simulink model "AcasXuClosedLoop_all_comp"  (Simulink 10.0)
settings: Solver=VariableStepAuto  StartTime=0.0  StopTime=20  RelTol=1e-3
libraries: neural x14040, simulink x2
(part 32 of 534)

== system AcasXuClosedLoop_all_comp/networks/Networks_m_7/AcasXu_5_7/Process Output 1 ==
  a : Inport  PortDimensions=5
      mask Display: e = 0.06;c1 = [120 230 180]/255;c2 = ([120 230 180]+0)/(256+128);c3 = ([120 230 180]+128)/(256+128);patch([0 1 1 0],[0 0 1 1],c1);patch([0 1 1 1-e 1-e 0+e],[0 0 1 1-e 0+e 0+e],c2);patch([0 0 1 1-e 0+e 0+e],[0 1 1 1-e 1-e 0+e],c3);plot([0 1 1 0 0],[0 0 1 1 0]);
  y : Outport  PortDimensions=5
      mask Display: e = 0.06;c1 = [120 230 180]/255;c2 = ([120 230 180]+0)/(256+128);c3 = ([120 230 180]+128)/(256+128);patch([0 1 1 0],[0 0 1 1],c1);patch([0 1 1 1-e 1-e 0+e],[0 0 1 1-e 0+e 0+e],c2);patch([0 0 1 1-e 0+e 0+e],[0 1 1 1-e 1-e 0+e],c3);plot([0 1 1 0 0],[0 0 1 1 0]);
  wires:
  a/1 -> y/1

== system AcasXuClosedLoop_all_comp/networks/Networks_m_8/AcasXu_1_8/Layer 1 ==
  "p{1}" : Inport  PortDimensions=5
      mask Display: e = 0.06;c1 = [120 230 180]/255;c2 = ([120 230 180]+0)/(256+128);c3 = ([120 230 180]+128)/(256+128);patch([0 1 1 0],[0 0 1 1],c1);patch([0 1 1 1-e 1-e 0+e],[0 0 1 1-e 0+e 0+e],c2);patch([0 0 1 1-e 0+e 0+e],[0 1 1 1-e 1-e 0+e],c3);plot([0 1 1 0 0],[0 0 1 1 0]);
  Delays 1 : Subsystem
      mask Display: e = 0.06;c1 = [120 150 230]/255;c2 = ([120 150 230]+0)/383;c3 = ([120 150 230]+128)/383;patch([0 1 1 0],[0 0 1 1],c1);patch([0 1 1 1-e 1-e 0+e],[0 0 1 1-e 0+e 0+e],c2);patch([0 0 1 1-e 0+e 0+e],[0 1 1 1-e 1-e 0+e],c3);plot([0 1 1 0 0],[0 0 1 1 0]);disp('0');
  "IW{1,1}" : Subsystem
      mask Display: e = 0.06;c1 = [120 150 230]/255;c2 = ([120 150 230]+0)/383;c3 = ([120 150 230]+128)/383;patch([0 1 1 0],[0 0 1 1],c1);patch([0 1 1 1-e 1-e 0+e],[0 0 1 1-e 0+e 0+e],c2);patch([0 0 1 1-e 0+e 0+e],[0 1 1 1-e 1-e 0+e],c3);plot([0 1 1 0 0],[0 0 1 1 0]);disp('W');
  "b{1}" : Constant  Value=[0.061786899999999998767119535614256164990365505218505859375;-0.11233100000000000029398705692074145190417766571044921875;0.0576498000000000010434320074637071229517459869384765625;-0.0022952799999999998030453252084726045723073184490203857421875;-0.020411800000000000776534392343819490633904933929443359375;-0.033371199999999996699262538868424599058926105499267578125;-0.0168530999999999993310684232028506812639534473419189453125;0.285644999999999982254195174391497857868671417236328125;0.074333399999999993923438523779623210430145263671875;-0.0687670999999999976726172690177918411791324615478515625;-0.055837400000000002309175073378355591557919979095458984375;-0.198448000000000013276491017677471973001956939697265625;0.2412819999999999964757080306299030780792236328125;-0.02656630000000000102478026065000449307262897491455078125;-0.15976000000000001310951347477384842932224273681640625;0.06498429999999999484572299479623325169086456298828125;-0.007162080000000000355731000212244907743297517299652099609375;0.03126600000000000212363460150299943052232265472412109375;0.2376500000000000001332267629550187848508358001708984375;-0.1867330000000000100879304909540223889052867889404296875;-0.1600370000000000125783827797931735403835773468017578125;0.03336530000000000051318949090273235924541950225830078125;-0.03460370000000000112461151502429856918752193450927734375;0.1750379999999999991455723602484795264899730682373046875;0.198494000000000003769429213207331486046314239501953125;0.1275840000000000029611868512802175246179103851318359375;-0.1219679999999999930881955378936254419386386871337890625;-0.0104092999999999998028687997475572046823799610137939453125;-0.06807520000000000237605490838177502155303955078125;0.0711587999999999942790651630275533534586429595947265625;-0.33700799999999997424993125605396926403045654296875;0.052991000000000003378186619329426321201026439666748046875;-0.045602999999999997704502874285026337020099163055419921875;0.09369750000000000300648395068492391146719455718994140625;-0.296754000000000017767121107681305147707462310791015625;0.006969020000000000249207321445510388002730906009674072265625;0.09820389999999999675051043368512182496488094329833984375;0.08321330000000000393090004990881425328552722930908203125;0.0544125000000000025313084961453569121658802032470703125;-0.07752489999999999381596893499590805731713771820068359375;0.1517699999999999882493995073673431761562824249267578125;-0.2425900000000000000799360577730112709105014801025390625;-0.31018000000000001126210236179758794605731964111328125;-0.13916600000000001191580167869688011705875396728515625;-0.164519999999999999573674358543939888477325439453125;-0.225654999999999994475530229465221054852008819580078125;-0.0209797000000000004316103030532758566550910472869873046875;0.260533999999999987817744795393082313239574432373046875;-0.0220758999999999989960475232919634436257183551788330078125;0.01041410000000000078690387539381845272146165370941162109375]
      mask Display: e = 0.06;c1 = [120 150 230]/255;c2 = ([120 150 230]+0)/383;c3 = ([120 150 230]+128)/383;patch([0 1 1 0],[0 0 1 1],c1);patch([0 1 1 1-e 1-e 0+e],[0 0 1 1-e 0+e 0+e],c2);patch([0 0 1 1-e 0+e 0+e],[0 1 1 1-e 1-e 0+e],c3);plot([0 1 1 0 0],[0 0 1 1 0]);disp('b');
  netsum : Sum  Inputs=2  SaturateOnIntegerOverflow=on  InputSameDT=on  OutDataTypeStr=Inherit: Same as first input
      mask Display: e = 0.06;c1 = [120 150 230]/255;c2 = ([120 150 230]+0)/383;c3 = ([120 150 230]+128)/383;patch([0 1 1 0],[0 0 1 1],c1);patch([0 1 1 1-e 1-e 0+e],[0 0 1 1-e 0+e 0+e],c2);patch([0 0 1 1-e 0+e 0+e],[0 1 1 1-e 1-e 0+e],c3);plot([0 1 1 0 0],[0 0 1 1 0]);disp('+');
  poslin : neural/Transfer Functions/poslin
      settings: SourceProductName=Deep Learning Toolbox  SourceProductBaseCode=NN
  "a{1}" : Outport  InitialOutput=[0;0;0;0;0;0;0;0;0;0;0;0;0;0;0;0;0;0;0;0;0;0;0;0;0;0;0;0;0;0;0;0;0;0;0;0;0;0;0;0;0;0;0;0;0;0;0;0;0;0]
      mask Display: e = 0.06;c1 = [120 230 180]/255;c2 = ([120 230 180]+0)/(256+128);c3 = ([120 230 180]+128)/(256+128);patch([0 1 1 0],[0 0 1 1],c1);patch([0 1 1 1-e 1-e 0+e],[0 0 1 1-e 0+e 0+e],c2);patch([0 0 1 1-e 0+e 0+e],[0 1 1 1-e 1-e 0+e],c3);plot([0 1 1 0 0],[0 0 1 1 0]);
  wires:
  poslin/1 -> "a{1}"/1
  netsum/1 -> poslin/1
  "b{1}"/1 -> netsum/2
  "IW{1,1}"/1("iz{1,1}") -> netsum/1
  Delays 1/1("pd{1,1}") -> "IW{1,1}"/1("pd{1,1}")
  "p{1}"/1 -> Delays 1/1("p{1}")

== system AcasXuClosedLoop_all_comp/networks/Networks_m_8/AcasXu_1_8/Layer 2 ==
  "a{1} " : Inport  PortDimensions=50
      mask Display: e = 0.06;c1 = [120 230 180]/255;c2 = ([120 230 180]+0)/(256+128);c3 = ([120 230 180]+128)/(256+128);patch([0 1 1 0],[0 0 1 1],c1);patch([0 1 1 1-e 1-e 0+e],[0 0 1 1-e 0+e 0+e],c2);patch([0 0 1 1-e 0+e 0+e],[0 1 1 1-e 1-e 0+e],c3);plot([0 1 1 0 0],[0 0 1 1 0]);
  Delays 1 : Subsystem
      mask Display: e = 0.06;c1 = [120 150 230]/255;c2 = ([120 150 230]+0)/383;c3 = ([120 150 230]+128)/383;patch([0 1 1 0],[0 0 1 1],c1);patch([0 1 1 1-e 1-e 0+e],[0 0 1 1-e 0+e 0+e],c2);patch([0 0 1 1-e 0+e 0+e],[0 1 1 1-e 1-e 0+e],c3);plot([0 1 1 0 0],[0 0 1 1 0]);disp('0');
  "LW{2,1}" : Subsystem
      mask Display: e = 0.06;c1 = [120 150 230]/255;c2 = ([120 150 230]+0)/383;c3 = ([120 150 230]+128)/383;patch([0 1 1 0],[0 0 1 1],c1);patch([0 1 1 1-e 1-e 0+e],[0 0 1 1-e 0+e 0+e],c2);patch([0 0 1 1-e 0+e 0+e],[0 1 1 1-e 1-e 0+e],c3);plot([0 1 1 0 0],[0 0 1 1 0]);disp('W');
  "b{2}" : Constant  Value=[-0.0749834000000000056029847428362700156867504119873046875;-0.0581537000000000026123103680220083333551883697509765625;-0.11407599999999999684785478848425555042922496795654296875;0.18763900000000000023447910280083306133747100830078125;-0.0798383000000000009332978834208915941417217254638671875;-0.1385989999999999999769073610877967439591884613037109375;-0.00337053999999999999437338971119970665313303470611572265625;-0.07958490000000000008650857807879219762980937957763671875;0.08194490000000000107238662394593120552599430084228515625;-0.05824000000000000010214051826551440171897411346435546875;-0.0244541999999999988546495188757035066373646259307861328125;-0.0194393999999999990413446226966698304750025272369384765625;0.0795817000000000052128967809039750136435031890869140625;-0.0909110999999999946918904925041715614497661590576171875;-0.360864999999999991331378623726777732372283935546875;-0.01107250000000000074440453801116746035404503345489501953125;-0.0245453999999999984693577204097891808487474918365478515625;0.0548796000000000006036060540282051078975200653076171875;-0.15333099999999999507593884118250571191310882568359375;0.005317529999999999999749089596434714621864259243011474609375;-0.1974700000000000066346927951599354855716228485107421875;0.3379440000000000221547225009999237954616546630859375;0.1953749999999999931166172473240294493734836578369140625;0.1484379999999999866222566424767137505114078521728515625;-0.11870799999999999407851447585926507599651813507080078125;0.358821000000000001062261389961349777877330780029296875;-0.19837199999999999278088580467738211154937744140625;-0.00835240999999999934877425999957267777062952518463134765625;0.0180682999999999989448884463172362302429974079132080078125;-0.2016679999999999861604038642326486296951770782470703125;-0.0012911699999999999045885434867386720725335180759429931640625;-0.00813783000000000041052050647749638301320374011993408203125;0.1612079999999999901927338896712171845138072967529296875;0.11871299999999999907895897877097013406455516815185546875;-0.15576699999999998880184648442082107067108154296875;0.1397279999999999910986758777653449214994907379150390625;0.1707849999999999923705473747759242542088031768798828125;-0.01066319999999999941497907940402001258917152881622314453125;0.1344269999999999909423564758981228806078433990478515625;-0.157517999999999991356247619478381238877773284912109375;-0.1907790000000000041335823652843828313052654266357421875;-0.000716729000000000042273906597500854331883601844310760498046875;0.16309899999999999398170302811195142567157745361328125;-0.09106720000000000092565954901147051714360713958740234375;-0.12074200000000000210231831943019642494618892669677734375;-0.0095464799999999995494537330387174733914434909820556640625;0.01164749999999999986732834855729379341937601566314697265625;-0.11863200000000000133848487848808872513473033905029296875;-0.1474350000000000104893871366584789939224720001220703125;-0.06965680000000000482440754012714023701846599578857421875]
      mask Display: e = 0.06;c1 = [120 150 230]/255;c2 = ([120 150 230]+0)/383;c3 = ([120 150 230]+128)/383;patch([0 1 1 0],[0 0 1 1],c1);patch([0 1 1 1-e 1-e 0+e],[0 0 1 1-e 0+e 0+e],c2);patch([0 0 1 1-e 0+e 0+e],[0 1 1 1-e 1-e 0+e],c3);plot([0 1 1 0 0],[0 0 1 1 0]);disp('b');
  netsum : Sum  Inputs=2  SaturateOnIntegerOverflow=on  InputSameDT=on  OutDataTypeStr=Inherit: Same as first input
      mask Display: e = 0.06;c1 = [120 150 230]/255;c2 = ([120 150 230]+0)/383;c3 = ([120 150 230]+128)/383;patch([0 1 1 0],[0 0 1 1],c1);patch([0 1 1 1-e 1-e 0+e],[0 0 1 1-e 0+e 0+e],c2);patch([0 0 1 1-e 0+e 0+e],[0 1 1 1-e 1-e 0+e],c3);plot([0 1 1 0 0],[0 0 1 1 0]);disp('+');
  poslin : neural/Transfer Functions/poslin
      settings: SourceProductName=Deep Learning Toolbox  SourceProductBaseCode=NN
  "a{2}" : Outport  InitialOutput=[0;0;0;0;0;0;0;0;0;0;0;0;0;0;0;0;0;0;0;0;0;0;0;0;0;0;0;0;0;0;0;0;0;0;0;0;0;0;0;0;0;0;0;0;0;0;0;0;0;0]
      mask Display: e = 0.06;c1 = [120 230 180]/255;c2 = ([120 230 180]+0)/(256+128);c3 = ([120 230 180]+128)/(256+128);patch([0 1 1 0],[0 0 1 1],c1);patch([0 1 1 1-e 1-e 0+e],[0 0 1 1-e 0+e 0+e],c2);patch([0 0 1 1-e 0+e 0+e],[0 1 1 1-e 1-e 0+e],c3);plot([0 1 1 0 0],[0 0 1 1 0]);
  wires:
  poslin/1 -> "a{2}"/1
  netsum/1 -> poslin/1
  "b{2}"/1 -> netsum/2
  "LW{2,1}"/1("lz{2,1}") -> netsum/1
  Delays 1/1("pd{2,1}") -> "LW{2,1}"/1("ad{2,1}")
  "a{1} "/1 -> Delays 1/1("p{2}")

== system AcasXuClosedLoop_all_comp/networks/Networks_m_8/AcasXu_1_8/Layer 3 ==
  "a{2} " : Inport  PortDimensions=50
      mask Display: e = 0.06;c1 = [120 230 180]/255;c2 = ([120 230 180]+0)/(256+128);c3 = ([120 230 180]+128)/(256+128);patch([0 1 1 0],[0 0 1 1],c1);patch([0 1 1 1-e 1-e 0+e],[0 0 1 1-e 0+e 0+e],c2);patch([0 0 1 1-e 0+e 0+e],[0 1 1 1-e 1-e 0+e],c3);plot([0 1 1 0 0],[0 0 1 1 0]);
  Delays 1 : Subsystem
      mask Display: e = 0.06;c1 = [120 150 230]/255;c2 = ([120 150 230]+0)/383;c3 = ([120 150 230]+128)/383;patch([0 1 1 0],[0 0 1 1],c1);patch([0 1 1 1-e 1-e 0+e],[0 0 1 1-e 0+e 0+e],c2);patch([0 0 1 1-e 0+e 0+e],[0 1 1 1-e 1-e 0+e],c3);plot([0 1 1 0 0],[0 0 1 1 0]);disp('0');
  "LW{3,2}" : Subsystem
      mask Display: e = 0.06;c1 = [120 150 230]/255;c2 = ([120 150 230]+0)/383;c3 = ([120 150 230]+128)/383;patch([0 1 1 0],[0 0 1 1],c1);patch([0 1 1 1-e 1-e 0+e],[0 0 1 1-e 0+e 0+e],c2);patch([0 0 1 1-e 0+e 0+e],[0 1 1 1-e 1-e 0+e],c3);plot([0 1 1 0 0],[0 0 1 1 0]);disp('W');
  "b{3}" : Constant  Value=[0.164017999999999997129407347529195249080657958984375;-0.26457399999999997586286326622939668595790863037109375;0.004655560000000000427100577127248470787890255451202392578125;-0.057429500000000001380673353423844673670828342437744140625;-0.013838599999999999512478865426601259969174861907958984375;-0.11519500000000000572430991496730712242424488067626953125;-0.06895700000000000440270042645352077670395374298095703125;0.06591560000000000474340566825048881582915782928466796875;-0.51487899999999997557864617192535661160945892333984375;0.1239139999999999963709029771052883006632328033447265625;0.049783000000000000861977156318971537984907627105712890625;-0.12134399999999999353317292616338818334043025970458984375;-0.5102510000000000101039177025086246430873870849609375;0.0264384000000000007890577080615912564098834991455078125;-0.450558999999999987284837743573007173836231231689453125;0.2221019999999999938733452609085361473262310028076171875;0.053726200000000001733013732518884353339672088623046875;-0.0053231800000000002726086023585594375617802143096923828125;-0.211625000000000007549516567451064474880695343017578125;0.0110915000000000006641354133307686424814164638519287109375;-0.01270549999999999978006481882175648934207856655120849609375;-0.180653000000000008018474773052730597555637359619140625;-0.2372209999999999874287226475644274614751338958740234375;0.246431000000000011151968237754772417247295379638671875;-0.320321000000000022378543462764355354011058807373046875;-0.1320280000000000064641625385775114409625530242919921875;-0.006335580000000000377990971855979296378791332244873046875;0.30925999999999997935873352616908960044384002685546875;-0.3744279999999999830606611794792115688323974609375;-0.0164010999999999983189891139545579790137708187103271484375;-0.2392469999999999874518152864766307175159454345703125;-0.1398790000000000033342217875542701222002506256103515625;0.068302899999999999725019961260841228067874908447265625;0.007190509999999999783681925435985249350778758525848388671875;-0.8442629999999999856896693017915822565555572509765625;-0.08072079999999999533333294721160200424492359161376953125;0.04693909999999999749231704981866641901433467864990234375;0.261462000000000027721824835680308751761913299560546875;0.2290979999999999960902385964800487272441387176513671875;0.00794875999999999915957005214295350015163421630859375;0.11019299999999999928324001530199893750250339508056640625;-0.2321689999999999864943589500398957170546054840087890625;-0.09204120000000000356710216919964295811951160430908203125;-0.0794713999999999975987208244987414218485355377197265625;0.18026500000000000856203996590920723974704742431640625;-0.244169999999999998152588887023739516735076904296875;-0.01446960000000000086395335330280431662686169147491455078125;-0.2047589999999999965663022294393158517777919769287109375;-0.338233999999999979113596282331855036318302154541015625;0.0191815000000000006330491686412642593495547771453857421875]
      mask Display: e = 0.06;c1 = [120 150 230]/255;c2 = ([120 150 230]+0)/383;c3 = ([120 150 230]+128)/383;patch([0 1 1 0],[0 0 1 1],c1);patch([0 1 1 1-e 1-e 0+e],[0 0 1 1-e 0+e 0+e],c2);patch([0 0 1 1-e 0+e 0+e],[0 1 1 1-e 1-e 0+e],c3);plot([0 1 1 0 0],[0 0 1 1 0]);disp('b');
  netsum : Sum  Inputs=2  SaturateOnIntegerOverflow=on  InputSameDT=on  OutDataTypeStr=Inherit: Same as first input
      mask Display: e = 0.06;c1 = [120 150 230]/255;c2 = ([120 150 230]+0)/383;c3 = ([120 150 230]+128)/383;patch([0 1 1 0],[0 0 1 1],c1);patch([0 1 1 1-e 1-e 0+e],[0 0 1 1-e 0+e 0+e],c2);patch([0 0 1 1-e 0+e 0+e],[0 1 1 1-e 1-e 0+e],c3);plot([0 1 1 0 0],[0 0 1 1 0]);disp('+');
  poslin : neural/Transfer Functions/poslin
      settings: SourceProductName=Deep Learning Toolbox  SourceProductBaseCode=NN
  "a{3}" : Outport  InitialOutput=[0;0;0;0;0;0;0;0;0;0;0;0;0;0;0;0;0;0;0;0;0;0;0;0;0;0;0;0;0;0;0;0;0;0;0;0;0;0;0;0;0;0;0;0;0;0;0;0;0;0]
      mask Display: e = 0.06;c1 = [120 230 180]/255;c2 = ([120 230 180]+0)/(256+128);c3 = ([120 230 180]+128)/(256+128);patch([0 1 1 0],[0 0 1 1],c1);patch([0 1 1 1-e 1-e 0+e],[0 0 1 1-e 0+e 0+e],c2);patch([0 0 1 1-e 0+e 0+e],[0 1 1 1-e 1-e 0+e],c3);plot([0 1 1 0 0],[0 0 1 1 0]);
  wires:
  poslin/1 -> "a{3}"/1
  netsum/1 -> poslin/1
  "b{3}"/1 -> netsum/2
  "LW{3,2}"/1("lz{3,2}") -> netsum/1
  Delays 1/1("pd{3,2}") -> "LW{3,2}"/1("ad{3,2}")
  "a{2} "/1 -> Delays 1/1("p{3}")

== system AcasXuClosedLoop_all_comp/networks/Networks_m_8/AcasXu_1_8/Layer 4 ==
  "a{3} " : Inport  PortDimensions=50
      mask Display: e = 0.06;c1 = [120 230 180]/255;c2 = ([120 230 180]+0)/(256+128);c3 = ([120 230 180]+128)/(256+128);patch([0 1 1 0],[0 0 1 1],c1);patch([0 1 1 1-e 1-e 0+e],[0 0 1 1-e 0+e 0+e],c2);patch([0 0 1 1-e 0+e 0+e],[0 1 1 1-e 1-e 0+e],c3);plot([0 1 1 0 0],[0 0 1 1 0]);
  Delays 1 : Subsystem
      mask Display: e = 0.06;c1 = [120 150 230]/255;c2 = ([120 150 230]+0)/383;c3 = ([120 150 230]+128)/383;patch([0 1 1 0],[0 0 1 1],c1);patch([0 1 1 1-e 1-e 0+e],[0 0 1 1-e 0+e 0+e],c2);patch([0 0 1 1-e 0+e 0+e],[0 1 1 1-e 1-e 0+e],c3);plot([0 1 1 0 0],[0 0 1 1 0]);disp('0');
  "LW{4,3}" : Subsystem
      mask Display: e = 0.06;c1 = [120 150 230]/255;c2 = ([120 150 230]+0)/383;c3 = ([120 150 230]+128)/383;patch([0 1 1 0],[0 0 1 1],c1);patch([0 1 1 1-e 1-e 0+e],[0 0 1 1-e 0+e 0+e],c2);patch([0 0 1 1-e 0+e 0+e],[0 1 1 1-e 1-e 0+e],c3);plot([0 1 1 0 0],[0 0 1 1 0]);disp('W');
  "b{4}" : Constant  Value=[0.0803641999999999967485564411617815494537353515625;-0.388384000000000007002398660915787331759929656982421875;-0.215119000000000004657607632907456718385219573974609375;-0.0056404400000000000370281583172982209362089633941650390625;-0.253674000000000010590639476504293270409107208251953125;-0.0938087999999999977429609998580417595803737640380859375;0.10869099999999999595079458458712906576693058013916015625;-0.44623800000000002352606998101691715419292449951171875;-0.78529999999999999804600747665972448885440826416015625;-0.1841790000000000093738350415151217021048069000244140625;-0.01542360000000000085085272161222746944986283779144287109375;-0.9974060000000000147934997585252858698368072509765625;0.06956199999999999883382173493373556993901729583740234375;0.006759560000000000394571042505731384153477847576141357421875;-0.003976249999999999749922263703183489269576966762542724609375;-0.009154189999999999549640250506854499690234661102294921875;-0.3285080000000000222115659198607318103313446044921875;-0.202494000000000007322142892007832415401935577392578125;-0.033443100000000003380051794010796584188938140869140625;-0.309585999999999972320807728465297259390354156494140625;-0.0287232000000000008144152019440298317931592464447021484375;-0.314433000000000018037127347270143218338489532470703125;0.06971860000000000556763524173220503143966197967529296875;0.038075400000000002298516932341954088769853115081787109375;0.09091749999999999831690189466826268471777439117431640625;0.1640060000000000128839161561700166203081607818603515625;-0.05116210000000000202025063344990485347807407379150390625;-0.016451000000000000345057316053498652763664722442626953125;0.291296999999999972619235677484539337456226348876953125;-0.0249990999999999997938093798666159273125231266021728515625;0.67291500000000004089173444299376569688320159912109375;0.346930000000000016147083670148276723921298980712890625;-0.310043999999999986272314345114864408969879150390625;-0.11016800000000000203659311637238715775310993194580078125;-0.5892779999999999684945350963971577584743499755859375;-0.218150000000000010569323194431490264832973480224609375;0.0703870000000000051176840543121215887367725372314453125;-0.2905590000000000117097442853264510631561279296875;-0.04409849999999999881072909602153231389820575714111328125;-0.190568999999999988403942552395164966583251953125;-0.1702470000000000094342311740547302179038524627685546875;0.183300999999999991718624414716032333672046661376953125;0.009201120000000000132001076735832612030208110809326171875;-0.2973259999999999791953086969442665576934814453125;-0.13172200000000000574829073229921050369739532470703125;-0.85807299999999997464072976072202436625957489013671875;-0.0139180999999999992222665667895853403024375438690185546875;-0.11071599999999999497379832291699131019413471221923828125;-0.416088999999999986645349281388917006552219390869140625;0.0796549000000000007037925797703792341053485870361328125]
      mask Display: e = 0.06;c1 = [120 150 230]/255;c2 = ([120 150 230]+0)/383;c3 = ([120 150 230]+128)/383;patch([0 1 1 0],[0 0 1 1],c1);patch([0 1 1 1-e 1-e 0+e],[0 0 1 1-e 0+e 0+e],c2);patch([0 0 1 1-e 0+e 0+e],[0 1 1 1-e 1-e 0+e],c3);plot([0 1 1 0 0],[0 0 1 1 0]);disp('b');
  netsum : Sum  Inputs=2  SaturateOnIntegerOverflow=on  InputSameDT=on  OutDataTypeStr=Inherit: Same as first input
      mask Display: e = 0.06;c1 = [120 150 230]/255;c2 = ([120 150 230]+0)/383;c3 = ([120 150 230]+128)/383;patch([0 1 1 0],[0 0 1 1],c1);patch([0 1 1 1-e 1-e 0+e],[0 0 1 1-e 0+e 0+e],c2);patch([0 0 1 1-e 0+e 0+e],[0 1 1 1-e 1-e 0+e],c3);plot([0 1 1 0 0],[0 0 1 1 0]);disp('+');
  poslin : neural/Transfer Functions/poslin
      settings: SourceProductName=Deep Learning Toolbox  SourceProductBaseCode=NN
  "a{4}" : Outport  InitialOutput=[0;0;0;0;0;0;0;0;0;0;0;0;0;0;0;0;0;0;0;0;0;0;0;0;0;0;0;0;0;0;0;0;0;0;0;0;0;0;0;0;0;0;0;0;0;0;0;0;0;0]
      mask Display: e = 0.06;c1 = [120 230 180]/255;c2 = ([120 230 180]+0)/(256+128);c3 = ([120 230 180]+128)/(256+128);patch([0 1 1 0],[0 0 1 1],c1);patch([0 1 1 1-e 1-e 0+e],[0 0 1 1-e 0+e 0+e],c2);patch([0 0 1 1-e 0+e 0+e],[0 1 1 1-e 1-e 0+e],c3);plot([0 1 1 0 0],[0 0 1 1 0]);
  wires:
  poslin/1 -> "a{4}"/1
  netsum/1 -> poslin/1
  "b{4}"/1 -> netsum/2
  "LW{4,3}"/1("lz{4,3}") -> netsum/1
  Delays 1/1("pd{4,3}") -> "LW{4,3}"/1("ad{4,3}")
  "a{3} "/1 -> Delays 1/1("p{4}")

== system AcasXuClosedLoop_all_comp/networks/Networks_m_8/AcasXu_1_8/Layer 5 ==
  "a{4} " : Inport  PortDimensions=50
      mask Display: e = 0.06;c1 = [120 230 180]/255;c2 = ([120 230 180]+0)/(256+128);c3 = ([120 230 180]+128)/(256+128);patch([0 1 1 0],[0 0 1 1],c1);patch([0 1 1 1-e 1-e 0+e],[0 0 1 1-e 0+e 0+e],c2);patch([0 0 1 1-e 0+e 0+e],[0 1 1 1-e 1-e 0+e],c3);plot([0 1 1 0 0],[0 0 1 1 0]);
  Delays 1 : Subsystem
      mask Display: e = 0.06;c1 = [120 150 230]/255;c2 = ([120 150 230]+0)/383;c3 = ([120 150 230]+128)/383;patch([0 1 1 0],[0 0 1 1],c1);patch([0 1 1 1-e 1-e 0+e],[0 0 1 1-e 0+e 0+e],c2);patch([0 0 1 1-e 0+e 0+e],[0 1 1 1-e 1-e 0+e],c3);plot([0 1 1 0 0],[0 0 1 1 0]);disp('0');
  "LW{5,4}" : Subsystem
      mask Display: e = 0.06;c1 = [120 150 230]/255;c2 = ([120 150 230]+0)/383;c3 = ([120 150 230]+128)/383;patch([0 1 1 0],[0 0 1 1],c1);patch([0 1 1 1-e 1-e 0+e],[0 0 1 1-e 0+e 0+e],c2);patch([0 0 1 1-e 0+e 0+e],[0 1 1 1-e 1-e 0+e],c3);plot([0 1 1 0 0],[0 0 1 1 0]);disp('W');
  "b{5}" : Constant  Value=[-0.11599099999999999688338192527226055972278118133544921875;-0.0257593999999999982708498436068111914210021495819091796875;-0.649186999999999958532725941040553152561187744140625;-0.03698769999999999835704755923870834521949291229248046875;0.042591400000000001480060518588288687169551849365234375;-0.61492599999999997262278839116333983838558197021484375;-1.3971400000000000485300688524148426949977874755859375;-0.426510000000000000230926389122032560408115386962890625;1.3822799999999999531752337134093977510929107666015625;-0.34164899999999998048139104867004789412021636962890625;-0.00423579999999999963489205612177102011628448963165283203125;-0.0239681999999999985340171093639582977630198001861572265625;-0.45559300000000002572875246187322773039340972900390625;0.1684039999999999981383780323085375130176544189453125;0.098771100000000000562749846721999347209930419921875;0.386554000000000008707701226740027777850627899169921875;-0.2345810000000000117292842105598538182675838470458984375;-0.1482489999999999918944837418166571296751499176025390625;-0.04539379999999999804050077045758371241390705108642578125;-0.0265822999999999996789679101993897347711026668548583984375;-0.0274131999999999986794563255898538045585155487060546875;-0.4334759999999999724451527072233147919178009033203125;0.03919419999999999848494525167552637867629528045654296875;-0.01862830000000000041371350789631833322346210479736328125;0.11089400000000000645616893280021031387150287628173828125;0.156901000000000012679635119638987816870212554931640625;-0.80331699999999994776800349427503533661365509033203125;0.09837569999999999648121473683204385451972484588623046875;-0.1727160000000000084074969208813854493200778961181640625;0.1573299999999999976285636194006656296551227569580078125;0.055510200000000002595879067257556016556918621063232421875;-0.355922000000000016139978242790675722062587738037109375;0.322979999999999989324095395204494707286357879638671875;-1.091979999999999950688334138249047100543975830078125;-0.254817000000000015713652601334615610539913177490234375;-0.047919499999999996819877168263701605610549449920654296875;-0.0198884000000000005226041821515536867082118988037109375;0.1737229999999999885407220290289842523634433746337890625;-0.2058629999999999904414238471872522495687007904052734375;-1.7048099999999999365485336966230534017086029052734375;-0.08241510000000000502051733519692788831889629364013671875;-0.91774900000000003696953854159801267087459564208984375;-2.30046000000000017138290786533616483211517333984375;-0.76807999999999998497202113867388106882572174072265625;-2.44972000000000011965539670200087130069732666015625;-0.1335469999999999990425436635632649995386600494384765625;-0.36733599999999999585753585051861591637134552001953125;0.1573279999999999956283858182359836064279079437255859375;0.09136640000000000039204195445563527755439281463623046875;-0.15914200000000000567723645872320048511028289794921875]
      mask Display: e = 0.06;c1 = [120 150 230]/255;c2 = ([120 150 230]+0)/383;c3 = ([120 150 230]+128)/383;patch([0 1 1 0],[0 0 1 1],c1);patch([0 1 1 1-e 1-e 0+e],[0 0 1 1-e 0+e 0+e],c2);patch([0 0 1 1-e 0+e 0+e],[0 1 1 1-e 1-e 0+e],c3);plot([0 1 1 0 0],[0 0 1 1 0]);disp('b');
  netsum : Sum  Inputs=2  SaturateOnIntegerOverflow=on  InputSameDT=on  OutDataTypeStr=Inherit: Same as first input
      mask Display: e = 0.06;c1 = [120 150 230]/255;c2 = ([120 150 230]+0)/383;c3 = ([120 150 230]+128)/383;patch([0 1 1 0],[0 0 1 1],c1);patch([0 1 1 1-e 1-e 0+e],[0 0 1 1-e 0+e 0+e],c2);patch([0 0 1 1-e 0+e 0+e],[0 1 1 1-e 1-e 0+e],c3);plot([0 1 1 0 0],[0 0 1 1 0]);disp('+');
  poslin : neural/Transfer Functions/poslin
      settings: SourceProductName=Deep Learning Toolbox  SourceProductBaseCode=NN
  "a{5}" : Outport  InitialOutput=[0;0;0;0;0;0;0;0;0;0;0;0;0;0;0;0;0;0;0;0;0;0;0;0;0;0;0;0;0;0;0;0;0;0;0;0;0;0;0;0;0;0;0;0;0;0;0;0;0;0]
      mask Display: e = 0.06;c1 = [120 230 180]/255;c2 = ([120 230 180]+0)/(256+128);c3 = ([120 230 180]+128)/(256+128);patch([0 1 1 0],[0 0 1 1],c1);patch([0 1 1 1-e 1-e 0+e],[0 0 1 1-e 0+e 0+e],c2);patch([0 0 1 1-e 0+e 0+e],[0 1 1 1-e 1-e 0+e],c3);plot([0 1 1 0 0],[0 0 1 1 0]);
  wires:
  poslin/1 -> "a{5}"/1
  netsum/1 -> poslin/1
  "b{5}"/1 -> netsum/2
  "LW{5,4}"/1("lz{5,4}") -> netsum/1
  Delays 1/1("pd{5,4}") -> "LW{5,4}"/1("ad{5,4}")
  "a{4} "/1 -> Delays 1/1("p{5}")

== system AcasXuClosedLoop_all_comp/networks/Networks_m_8/AcasXu_1_8/Layer 6 ==
  "a{5} " : Inport  PortDimensions=50
      mask Display: e = 0.06;c1 = [120 230 180]/255;c2 = ([120 230 180]+0)/(256+128);c3 = ([120 230 180]+128)/(256+128);patch([0 1 1 0],[0 0 1 1],c1);patch([0 1 1 1-e 1-e 0+e],[0 0 1 1-e 0+e 0+e],c2);patch([0 0 1 1-e 0+e 0+e],[0 1 1 1-e 1-e 0+e],c3);plot([0 1 1 0 0],[0 0 1 1 0]);
  Delays 1 : Subsystem
      mask Display: e = 0.06;c1 = [120 150 230]/255;c2 = ([120 150 230]+0)/383;c3 = ([120 150 230]+128)/383;patch([0 1 1 0],[0 0 1 1],c1);patch([0 1 1 1-e 1-e 0+e],[0 0 1 1-e 0+e 0+e],c2);patch([0 0 1 1-e 0+e 0+e],[0 1 1 1-e 1-e 0+e],c3);plot([0 1 1 0 0],[0 0 1 1 0]);disp('0');
  "LW{6,5}" : Subsystem
      mask Display: e = 0.06;c1 = [120 150 230]/255;c2 = ([120 150 230]+0)/383;c3 = ([120 150 230]+128)/383;patch([0 1 1 0],[0 0 1 1],c1);patch([0 1 1 1-e 1-e 0+e],[0 0 1 1-e 0+e 0+e],c2);patch([0 0 1 1-e 0+e 0+e],[0 1 1 1-e 1-e 0+e],c3);plot([0 1 1 0 0],[0 0 1 1 0]);disp('W');
  "b{6}" : Constant  Value=[0.1032160000000000021902479829805088229477405548095703125;-0.333413000000000014910739309925702400505542755126953125;0.034123199999999999365574154808200546540319919586181640625;-0.0192576999999999991242116692546915146522223949432373046875;-0.018888599999999998446664761786450981162488460540771484375;0.05005020000000000301998426266436581499874591827392578125;0.0900260999999999977916331772576086223125457763671875;0.65171999999999996600053009387920610606670379638671875;0.25178299999999997904609472243464551866054534912109375;-0.06583709999999999562536601160900318063795566558837890625;-0.03132859999999999811581830044815433211624622344970703125;-0.07531680000000000319015924787890980951488018035888671875;-1.14986999999999994770405464805662631988525390625;-0.91863399999999995060306900995783507823944091796875;0.233418999999999987604581974665052257478237152099609375;0.01594500000000000083932860661661834456026554107666015625;0.0306607999999999984719334378269195440225303173065185546875;-0.647046000000000010032863428932614624500274658203125;0.351486000000000020637713760152109898626804351806640625;-0.047706400000000002969979817635248764418065547943115234375;0.275490000000000012647660696529783308506011962890625;-0.0678615999999999941483252996476949192583560943603515625;-0.382317999999999991178611935538356192409992218017578125;-0.0031887500000000001780520175742594801704399287700653076171875;-1.204590000000000049595882956054992973804473876953125;0.143919999999999992379429158972925506532192230224609375;0.447313000000000016154189097505877725780010223388671875;0.5213950000000000528643795405514538288116455078125;0.843636000000000052523319027386605739593505859375;-0.04831560000000000021369572777985013090074062347412109375;0.08911429999999999351789625734454602934420108795166015625;0.069440000000000001723066134218242950737476348876953125;-1.217449999999999921129756330628879368305206298828125;-0.1164779999999999982041032353663467802107334136962890625;-0.037601500000000002976729973624969716183841228485107421875;0.1009359999999999979447551368139102123677730560302734375;0.6564229999999999787263504913426004350185394287109375;0.1446659999999999890452073714186553843319416046142578125;-0.01508660000000000035724756486388287157751619815826416015625;0.155961999999999989530152788574923761188983917236328125;-0.02799190000000000011493028750919620506465435028076171875;0.133743000000000000770938868299708701670169830322265625;0.092265700000000006042455424903891980648040771484375;0.156538000000000010469847211425076238811016082763671875;0.84865500000000004821032462132279761135578155517578125;0.07894980000000000053272941613613511435687541961669921875;0.07541290000000000492974550070357508957386016845703125;0.1804840000000000055369042684105806984007358551025390625;-0.01051580000000000049420467718164218240417540073394775390625;0.0758058000000000065110583591376780532300472259521484375]
      mask Display: e = 0.06;c1 = [120 150 230]/255;c2 = ([120 150 230]+0)/383;c3 = ([120 150 230]+128)/383;patch([0 1 1 0],[0 0 1 1],c1);patch([0 1 1 1-e 1-e 0+e],[0 0 1 1-e 0+e 0+e],c2);patch([0 0 1 1-e 0+e 0+e],[0 1 1 1-e 1-e 0+e],c3);plot([0 1 1 0 0],[0 0 1 1 0]);disp('b');
  netsum : Sum  Inputs=2  SaturateOnIntegerOverflow=on  InputSameDT=on  OutDataTypeStr=Inherit: Same as first input
      mask Display: e = 0.06;c1 = [120 150 230]/255;c2 = ([120 150 230]+0)/383;c3 = ([120 150 230]+128)/383;patch([0 1 1 0],[0 0 1 1],c1);patch([0 1 1 1-e 1-e 0+e],[0 0 1 1-e 0+e 0+e],c2);patch([0 0 1 1-e 0+e 0+e],[0 1 1 1-e 1-e 0+e],c3);plot([0 1 1 0 0],[0 0 1 1 0]);disp('+');
  poslin : neural/Transfer Functions/poslin
      settings: SourceProductName=Deep Learning Toolbox  SourceProductBaseCode=NN
  "a{6}" : Outport  InitialOutput=[0;0;0;0;0;0;0;0;0;0;0;0;0;0;0;0;0;0;0;0;0;0;0;0;0;0;0;0;0;0;0;0;0;0;0;0;0;0;0;0;0;0;0;0;0;0;0;0;0;0]
      mask Display: e = 0.06;c1 = [120 230 180]/255;c2 = ([120 230 180]+0)/(256+128);c3 = ([120 230 180]+128)/(256+128);patch([0 1 1 0],[0 0 1 1],c1);patch([0 1 1 1-e 1-e 0+e],[0 0 1 1-e 0+e 0+e],c2);patch([0 0 1 1-e 0+e 0+e],[0 1 1 1-e 1-e 0+e],c3);plot([0 1 1 0 0],[0 0 1 1 0]);
  wires:
  poslin/1 -> "a{6}"/1
  netsum/1 -> poslin/1
  "b{6}"/1 -> netsum/2
  "LW{6,5}"/1("lz{6,5}") -> netsum/1
  Delays 1/1("pd{6,5}") -> "LW{6,5}"/1("ad{6,5}")
  "a{5} "/1 -> Delays 1/1("p{6}")

== system AcasXuClosedLoop_all_comp/networks/Networks_m_8/AcasXu_1_8/Layer 7 ==
  "a{6} " : Inport  PortDimensions=50
      mask Display: e = 0.06;c1 = [120 230 180]/255;c2 = ([120 230 180]+0)/(256+128);c3 = ([120 230 180]+128)/(256+128);patch([0 1 1 0],[0 0 1 1],c1);patch([0 1 1 1-e 1-e 0+e],[0 0 1 1-e 0+e 0+e],c2);patch([0 0 1 1-e 0+e 0+e],[0 1 1 1-e 1-e 0+e],c3);plot([0 1 1 0 0],[0 0 1 1 0]);
  Delays 1 : Subsystem
      mask Display: e = 0.06;c1 = [120 150 230]/255;c2 = ([120 150 230]+0)/383;c3 = ([120 150 230]+128)/383;patch([0 1 1 0],[0 0 1 1],c1);patch([0 1 1 1-e 1-e 0+e],[0 0 1 1-e 0+e 0+e],c2);patch([0 0 1 1-e 0+e 0+e],[0 1 1 1-e 1-e 0+e],c3);plot([0 1 1 0 0],[0 0 1 1 0]);disp('0');
  "LW{7,6}" : Subsystem
      mask Display: e = 0.06;c1 = [120 150 230]/255;c2 = ([120 150 230]+0)/383;c3 = ([120 150 230]+128)/383;patch([0 1 1 0],[0 0 1 1],c1);patch([0 1 1 1-e 1-e 0+e],[0 0 1 1-e 0+e 0+e],c2);patch([0 0 1 1-e 0+e 0+e],[0 1 1 1-e 1-e 0+e],c3);plot([0 1 1 0 0],[0 0 1 1 0]);disp('W');
  "b{7}" : Constant  Value=[-0.020205700000000000049471537977296975441277027130126953125;-0.01944289999999999907220882278124918229877948760986328125;-0.0194251000000000006995293233558186329901218414306640625;-0.019471599999999998631405873084077029488980770111083984375;-0.01949309999999999931663552388272364623844623565673828125]
      mask Display: e = 0.06;c1 = [120 150 230]/255;c2 = ([120 150 230]+0)/383;c3 = ([120 150 230]+128)/383;patch([0 1 1 0],[0 0 1 1],c1);patch([0 1 1 1-e 1-e 0+e],[0 0 1 1-e 0+e 0+e],c2);patch([0 0 1 1-e 0+e 0+e],[0 1 1 1-e 1-e 0+e],c3);plot([0 1 1 0 0],[0 0 1 1 0]);disp('b');
  netsum : Sum  Inputs=2  SaturateOnIntegerOverflow=on  InputSameDT=on  OutDataTypeStr=Inherit: Same as first input
      mask Display: e = 0.06;c1 = [120 150 230]/255;c2 = ([120 150 230]+0)/383;c3 = ([120 150 230]+128)/383;patch([0 1 1 0],[0 0 1 1],c1);patch([0 1 1 1-e 1-e 0+e],[0 0 1 1-e 0+e 0+e],c2);patch([0 0 1 1-e 0+e 0+e],[0 1 1 1-e 1-e 0+e],c3);plot([0 1 1 0 0],[0 0 1 1 0]);disp('+');
  purelin : neural/Transfer Functions/purelin
      settings: SourceProductName=Deep Learning Toolbox
  "a{7}" : Outport  InitialOutput=[0;0;0;0;0]
      mask Display: e = 0.06;c1 = [120 230 180]/255;c2 = ([120 230 180]+0)/(256+128);c3 = ([120 230 180]+128)/(256+128);patch([0 1 1 0],[0 0 1 1],c1);patch([0 1 1 1-e 1-e 0+e],[0 0 1 1-e 0+e 0+e],c2);patch([0 0 1 1-e 0+e 0+e],[0 1 1 1-e 1-e 0+e],c3);plot([0 1 1 0 0],[0 0 1 1 0]);
  wires:
  purelin/1 -> "a{7}"/1
  netsum/1 -> purelin/1
  "b{7}"/1 -> netsum/2
  "LW{7,6}"/1("lz{7,6}") -> netsum/1
  Delays 1/1("pd{7,6}") -> "LW{7,6}"/1("ad{7,6}")
  "a{6} "/1 -> Delays 1/1("p{7}")

== system AcasXuClosedLoop_all_comp/networks/Networks_m_8/AcasXu_1_8/Process Input 1 ==
  x : Inport  PortDimensions=5
      mask Display: e = 0.06;c1 = [120 230 180]/255;c2 = ([120 230 180]+0)/(256+128);c3 = ([120 230 180]+128)/(256+128);patch([0 1 1 0],[0 0 1 1],c1);patch([0 1 1 1-e 1-e 0+e],[0 0 1 1-e 0+e 0+e],c2);patch([0 0 1 1-e 0+e 0+e],[0 1 1 1-e 1-e 0+e],c3);plot([0 1 1 0 0],[0 0 1 1 0]);
  p : Outport  PortDimensions=5
      mask Display: e = 0.06;c1 = [120 230 180]/255;c2 = ([120 230 180]+0)/(256+128);c3 = ([120 230 180]+128)/(256+128);patch([0 1 1 0],[0 0 1 1],c1);patch([0 1 1 1-e 1-e 0+e],[0 0 1 1-e 0+e 0+e],c2);patch([0 0 1 1-e 0+e 0+e],[0 1 1 1-e 1-e 0+e],c3);plot([0 1 1 0 0],[0 0 1 1 0]);
  wires:
  x/1 -> p/1

== system AcasXuClosedLoop_all_comp/networks/Networks_m_8/AcasXu_1_8/Process Output 1 ==
  a : Inport  PortDimensions=5
      mask Display: e = 0.06;c1 = [120 230 180]/255;c2 = ([120 230 180]+0)/(256+128);c3 = ([120 230 180]+128)/(256+128);patch([0 1 1 0],[0 0 1 1],c1);patch([0 1 1 1-e 1-e 0+e],[0 0 1 1-e 0+e 0+e],c2);patch([0 0 1 1-e 0+e 0+e],[0 1 1 1-e 1-e 0+e],c3);plot([0 1 1 0 0],[0 0 1 1 0]);
  y : Outport  PortDimensions=5
      mask Display: e = 0.06;c1 = [120 230 180]/255;c2 = ([120 230 180]+0)/(256+128);c3 = ([120 230 180]+128)/(256+128);patch([0 1 1 0],[0 0 1 1],c1);patch([0 1 1 1-e 1-e 0+e],[0 0 1 1-e 0+e 0+e],c2);patch([0 0 1 1-e 0+e 0+e],[0 1 1 1-e 1-e 0+e],c3);plot([0 1 1 0 0],[0 0 1 1 0]);
  wires:
  a/1 -> y/1

== system AcasXuClosedLoop_all_comp/networks/Networks_m_8/AcasXu_2_8/Layer 1 ==
  "p{1}" : Inport  PortDimensions=5
      mask Display: e = 0.06;c1 = [120 230 180]/255;c2 = ([120 230 180]+0)/(256+128);c3 = ([120 230 180]+128)/(256+128);patch([0 1 1 0],[0 0 1 1],c1);patch([0 1 1 1-e 1-e 0+e],[0 0 1 1-e 0+e 0+e],c2);patch([0 0 1 1-e 0+e 0+e],[0 1 1 1-e 1-e 0+e],c3);plot([0 1 1 0 0],[0 0 1 1 0]);
  Delays 1 : Subsystem
      mask Display: e = 0.06;c1 = [120 150 230]/255;c2 = ([120 150 230]+0)/383;c3 = ([120 150 230]+128)/383;patch([0 1 1 0],[0 0 1 1],c1);patch([0 1 1 1-e 1-e 0+e],[0 0 1 1-e 0+e 0+e],c2);patch([0 0 1 1-e 0+e 0+e],[0 1 1 1-e 1-e 0+e],c3);plot([0 1 1 0 0],[0 0 1 1 0]);disp('0');
  "IW{1,1}" : Subsystem
      mask Display: e = 0.06;c1 = [120 150 230]/255;c2 = ([120 150 230]+0)/383;c3 = ([120 150 230]+128)/383;patch([0 1 1 0],[0 0 1 1],c1);patch([0 1 1 1-e 1-e 0+e],[0 0 1 1-e 0+e 0+e],c2);patch([0 0 1 1-e 0+e 0+e],[0 1 1 1-e 1-e 0+e],c3);plot([0 1 1 0 0],[0 0 1 1 0]);disp('W');
  "b{1}" : Constant  Value=[0.0292530000000000012239098623467725701630115509033203125;-0.0192979999999999991822097200611096923239529132843017578125;0.043707400000000000417355039417088846676051616668701171875;-0.10806100000000000427302637717730249278247356414794921875;0.69227600000000000246558329308754764497280120849609375;-0.0055904900000000000426236823614090098999440670013427734375;-0.0137473000000000004916511642250043223612010478973388671875;-0.54575099999999998612310037060524336993694305419921875;0.12727399999999999824495944267255254089832305908203125;-0.404532999999999975937470253484207205474376678466796875;-0.2511010000000000186304305316298268735408782958984375;0.253942999999999974303221961235976777970790863037109375;-0.430788000000000004252598273524199612438678741455078125;0.0254458000000000010565326391542839701287448406219482421875;-0.09328599999999999392574778767084353603422641754150390625;-0.31926100000000001699618223938159644603729248046875;-0.0173319000000000007777334332104146596975624561309814453125;0.035675699999999997691357833673464483581483364105224609375;-0.199647999999999992137844628814491443336009979248046875;-0.1559500000000000052846615972157451324164867401123046875;-0.31858500000000000706990022081299684941768646240234375;-0.386705000000000020943247136528952978551387786865234375;-0.344754999999999978133047306982916779816150665283203125;-0.01401640000000000003954614413714807596988976001739501953125;-0.272656000000000009464429240324534475803375244140625;0.03867310000000000191899829360409057699143886566162109375;-0.2313759999999999983355536414819653145968914031982421875;-0.15332699999999999107558323885314166545867919921875;-0.03369239999999999735091904540240648202598094940185546875;0.1966759999999999897202229703907505609095096588134765625;-0.435016000000000013780976360067143104970455169677734375;-0.2584049999999999958077978590154089033603668212890625;-0.199925999999999992606802834416157566010951995849609375;-0.0253414999999999994428900862430964480154216289520263671875;-0.16625400000000001288213979933061636984348297119140625;-0.343000000000000027089441800853819586336612701416015625;-0.138017000000000000792255150372511707246303558349609375;0.1802249999999999963140595582444802857935428619384765625;-0.5465459999999999762820834803278557956218719482421875;-0.17718000000000000415667500419658608734607696533203125;-0.11433000000000000107025499573865090496838092803955078125;-0.54911699999999996624211462403764016926288604736328125;-0.722998000000000029530156098189763724803924560546875;-0.27104099999999997638866489069187082350254058837890625;-0.180599000000000009524825372864142991602420806884765625;-0.393623000000000000664357457935693673789501190185546875;-0.018489999999999999491517854721678304485976696014404296875;-0.044360900000000001719424602697472437284886837005615234375;-0.0236127999999999997615685032315013813786208629608154296875;0.1266879999999999950599516296279034577310085296630859375]
      mask Display: e = 0.06;c1 = [120 150 230]/255;c2 = ([120 150 230]+0)/383;c3 = ([120 150 230]+128)/383;patch([0 1 1 0],[0 0 1 1],c1);patch([0 1 1 1-e 1-e 0+e],[0 0 1 1-e 0+e 0+e],c2);patch([0 0 1 1-e 0+e 0+e],[0 1 1 1-e 1-e 0+e],c3);plot([0 1 1 0 0],[0 0 1 1 0]);disp('b');
  netsum : Sum  Inputs=2  SaturateOnIntegerOverflow=on  InputSameDT=on  OutDataTypeStr=Inherit: Same as first input
      mask Display: e = 0.06;c1 = [120 150 230]/255;c2 = ([120 150 230]+0)/383;c3 = ([120 150 230]+128)/383;patch([0 1 1 0],[0 0 1 1],c1);patch([0 1 1 1-e 1-e 0+e],[0 0 1 1-e 0+e 0+e],c2);patch([0 0 1 1-e 0+e 0+e],[0 1 1 1-e 1-e 0+e],c3);plot([0 1 1 0 0],[0 0 1 1 0]);disp('+');
  poslin : neural/Transfer Functions/poslin
      settings: SourceProductName=Deep Learning Toolbox  SourceProductBaseCode=NN
  "a{1}" : Outport  InitialOutput=[0;0;0;0;0;0;0;0;0;0;0;0;0;0;0;0;0;0;0;0;0;0;0;0;0;0;0;0;0;0;0;0;0;0;0;0;0;0;0;0;0;0;0;0;0;0;0;0;0;0]
      mask Display: e = 0.06;c1 = [120 230 180]/255;c2 = ([120 230 180]+0)/(256+128);c3 = ([120 230 180]+128)/(256+128);patch([0 1 1 0],[0 0 1 1],c1);patch([0 1 1 1-e 1-e 0+e],[0 0 1 1-e 0+e 0+e],c2);patch([0 0 1 1-e 0+e 0+e],[0 1 1 1-e 1-e 0+e],c3);plot([0 1 1 0 0],[0 0 1 1 0]);
  wires:
  poslin/1 -> "a{1}"/1
  netsum/1 -> poslin/1
  "b{1}"/1 -> netsum/2
  "IW{1,1}"/1("iz{1,1}") -> netsum/1
  Delays 1/1("pd{1,1}") -> "IW{1,1}"/1("pd{1,1}")
  "p{1}"/1 -> Delays 1/1("p{1}")

== system AcasXuClosedLoop_all_comp/networks/Networks_m_8/AcasXu_2_8/Layer 2 ==
  "a{1} " : Inport  PortDimensions=50
      mask Display: e = 0.06;c1 = [120 230 180]/255;c2 = ([120 230 180]+0)/(256+128);c3 = ([120 230 180]+128)/(256+128);patch([0 1 1 0],[0 0 1 1],c1);patch([0 1 1 1-e 1-e 0+e],[0 0 1 1-e 0+e 0+e],c2);patch([0 0 1 1-e 0+e 0+e],[0 1 1 1-e 1-e 0+e],c3);plot([0 1 1 0 0],[0 0 1 1 0]);
  Delays 1 : Subsystem
      mask Display: e = 0.06;c1 = [120 150 230]/255;c2 = ([120 150 230]+0)/383;c3 = ([120 150 230]+128)/383;patch([0 1 1 0],[0 0 1 1],c1);patch([0 1 1 1-e 1-e 0+e],[0 0 1 1-e 0+e 0+e],c2);patch([0 0 1 1-e 0+e 0+e],[0 1 1 1-e 1-e 0+e],c3);plot([0 1 1 0 0],[0 0 1 1 0]);disp('0');
  "LW{2,1}" : Subsystem
      mask Display: e = 0.06;c1 = [120 150 230]/255;c2 = ([120 150 230]+0)/383;c3 = ([120 150 230]+128)/383;patch([0 1 1 0],[0 0 1 1],c1);patch([0 1 1 1-e 1-e 0+e],[0 0 1 1-e 0+e 0+e],c2);patch([0 0 1 1-e 0+e 0+e],[0 1 1 1-e 1-e 0+e],c3);plot([0 1 1 0 0],[0 0 1 1 0]);disp('W');
  "b{2}" : Constant  Value=[-0.36615100000000000424194013248779810965061187744140625;0.416528999999999982595255687556345947086811065673828125;0.77459800000000000874678107720683328807353973388671875;-0.0122302000000000000989874848755789571441709995269775390625;-0.403969000000000022509993868879973888397216796875;-0.215281000000000000138555833473219536244869232177734375;-0.01143070000000000023543389460201069596223533153533935546875;-0.448421000000000014029666317583178170025348663330078125;0.740712999999999954781060296227224171161651611328125;-0.338034000000000001140421090894960798323154449462890625;-0.0179464000000000012402523452692548744380474090576171875;1.765390000000000014779288903810083866119384765625;-1.2149300000000000654409859635052271187305450439453125;0.91112099999999995869615077026537619531154632568359375;-0.0101702000000000007673417456999231944791972637176513671875;-0.0120105999999999997485122804619095404632389545440673828125;1.3918299999999999005950712671619839966297149658203125;0.0163710000000000001352251643993440666235983371734619140625;0.5273060000000000524522647538105957210063934326171875;0.44628800000000001801936377887614071369171142578125;-0.391253999999999990677679306827485561370849609375;-0.0160214000000000016121770585186823154799640178680419921875;-1.07111000000000000653699316899292171001434326171875;-0.10115200000000000579714054538271739147603511810302734375;-0.56880299999999994753352439147420227527618408203125;1.0061999999999999833022457096376456320285797119140625;-0.127674000000000009702461056804168038070201873779296875;0.61763800000000002032862767009646631777286529541015625;-0.1499989999999999934487959762918762862682342529296875;-0.2048290000000000110613740389453596435487270355224609375;0.7154700000000000503774799653911031782627105712890625;-0.11080099999999999671462802552923676557838916778564453125;0.213083999999999995633714888754184357821941375732421875;0.36989199999999999857180910112219862639904022216796875;-0.004811980000000000423643342628565733321011066436767578125;-0.1587030000000000107274189531381125561892986297607421875;-0.4675489999999999923829818726517260074615478515625;-0.0220813000000000016209700248737135552801191806793212890625;0.7037560000000000481890083392499946057796478271484375;0.4845479999999999787263504913426004350185394287109375;0.8998909999999999964614971759147010743618011474609375;-0.50484099999999998420463498405297286808490753173828125;-1.22276000000000006906475391588173806667327880859375;-0.1698209999999999997299937604111619293689727783203125;-0.0117032999999999998141930745987338013947010040283203125;0.1784880000000000077609030313396942801773548126220703125;-0.484412999999999982492226990871131420135498046875;-0.00656782000000000025508040124577746610157191753387451171875;-0.337096999999999979991116560995578765869140625;-0.007772699999999999574684661496348780929110944271087646484375]
      mask Display: e = 0.06;c1 = [120 150 230]/255;c2 = ([120 150 230]+0)/383;c3 = ([120 150 230]+128)/383;patch([0 1 1 0],[0 0 1 1],c1);patch([0 1 1 1-e 1-e 0+e],[0 0 1 1-e 0+e 0+e],c2);patch([0 0 1 1-e 0+e 0+e],[0 1 1 1-e 1-e 0+e],c3);plot([0 1 1 0 0],[0 0 1 1 0]);disp('b');
  netsum : Sum  Inputs=2  SaturateOnIntegerOverflow=on  InputSameDT=on  OutDataTypeStr=Inherit: Same as first input
      mask Display: e = 0.06;c1 = [120 150 230]/255;c2 = ([120 150 230]+0)/383;c3 = ([120 150 230]+128)/383;patch([0 1 1 0],[0 0 1 1],c1);patch([0 1 1 1-e 1-e 0+e],[0 0 1 1-e 0+e 0+e],c2);patch([0 0 1 1-e 0+e 0+e],[0 1 1 1-e 1-e 0+e],c3);plot([0 1 1 0 0],[0 0 1 1 0]);disp('+');
  poslin : neural/Transfer Functions/poslin
      settings: SourceProductName=Deep Learning Toolbox  SourceProductBaseCode=NN
  "a{2}" : Outport  InitialOutput=[0;0;0;0;0;0;0;0;0;0;0;0;0;0;0;0;0;0;0;0;0;0;0;0;0;0;0;0;0;0;0;0;0;0;0;0;0;0;0;0;0;0;0;0;0;0;0;0;0;0]
      mask Display: e = 0.06;c1 = [120 230 180]/255;c2 = ([120 230 180]+0)/(256+128);c3 = ([120 230 180]+128)/(256+128);patch([0 1 1 0],[0 0 1 1],c1);patch([0 1 1 1-e 1-e 0+e],[0 0 1 1-e 0+e 0+e],c2);patch([0 0 1 1-e 0+e 0+e],[0 1 1 1-e 1-e 0+e],c3);plot([0 1 1 0 0],[0 0 1 1 0]);
  wires:
  poslin/1 -> "a{2}"/1
  netsum/1 -> poslin/1
  "b{2}"/1 -> netsum/2
  "LW{2,1}"/1("lz{2,1}") -> netsum/1
  Delays 1/1("pd{2,1}") -> "LW{2,1}"/1("ad{2,1}")
  "a{1} "/1 -> Delays 1/1("p{2}")
